simulink library "R9000_FirmwareLib"  (R2024b)
settings: Solver=VariableStepAuto  StartTime=0.0  StopTime=10.0  RelTol=1e-3
libraries: mbd_s32k3_io_library x82, mbd_s32k3_isr_library x15, mbd_s32k3_communication_library x10, mbd_s32k3_utility_library x1
(part 2 of 7)

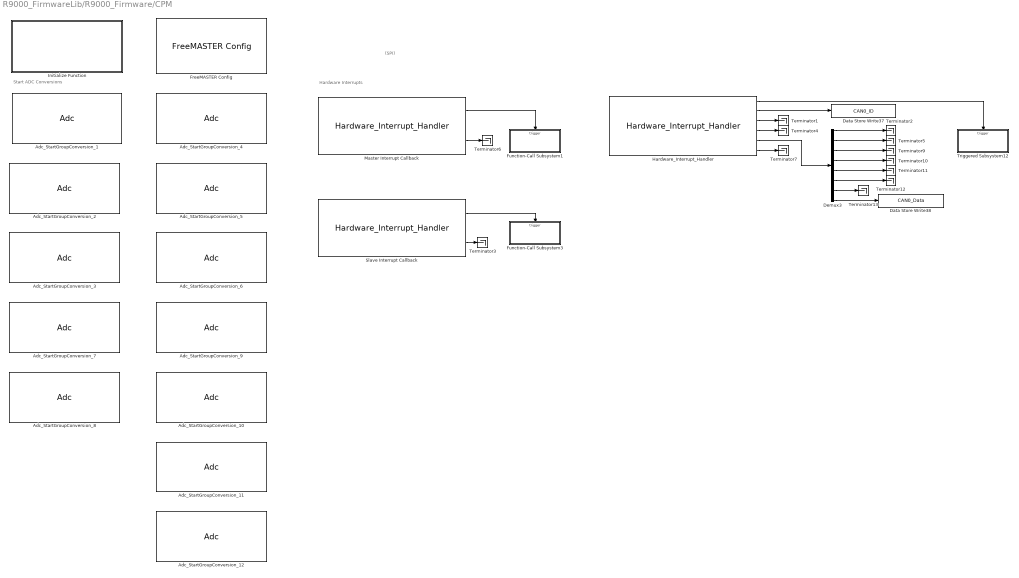
== system R9000_FirmwareLib/R9000_Firmware/CPM ==
  note: Start ADC Conversions
  note: Hardware Interrupts
  note: (SPI)
  Adc_StartGroupConversion_1 : mbd_s32k3_io_library/Adc  Priority=2  api_func=Adc_StartGroupConversion  hw_unit=AdcHwUnit_0  group=Adc0Group_0  channel=ADC_1_AI2  threshold_control=No ADC threshold control configured  power_state=ADC_FULL_POWER  ctu_unit=BctuHwUnit_0  ctu_trigger=AdcHwTrigger_0  ctu_fifo_idx=BctuResultFifos_0  text=<binary blob, 63510 chars, not shown>
      settings: SourceProductName=IO Blocks
  Adc_StartGroupConversion_10 : mbd_s32k3_io_library/Adc  Priority=2  api_func=Adc_StartGroupConversion  hw_unit=AdcHwUnit_0  group=Adc2Group_4  channel=ADC_1_AI2  threshold_control=No ADC threshold control configured  power_state=ADC_FULL_POWER  ctu_unit=BctuHwUnit_0  ctu_trigger=AdcHwTrigger_0  ctu_fifo_idx=BctuResultFifos_0  text=<binary blob, 63510 chars, not shown>
      settings: SourceProductName=IO Blocks
  Adc_StartGroupConversion_11 : mbd_s32k3_io_library/Adc  Priority=2  api_func=Adc_StartGroupConversion  hw_unit=AdcHwUnit_0  group=Adc2Group_5  channel=ADC_1_AI2  threshold_control=No ADC threshold control configured  power_state=ADC_FULL_POWER  ctu_unit=BctuHwUnit_0  ctu_trigger=AdcHwTrigger_0  ctu_fifo_idx=BctuResultFifos_0  text=<binary blob, 63510 chars, not shown>
      settings: SourceProductName=IO Blocks
  Adc_StartGroupConversion_12 : mbd_s32k3_io_library/Adc  Priority=2  api_func=Adc_StartGroupConversion  hw_unit=AdcHwUnit_0  group=Adc2Group_6  channel=ADC_1_AI2  threshold_control=No ADC threshold control configured  power_state=ADC_FULL_POWER  ctu_unit=BctuHwUnit_0  ctu_trigger=AdcHwTrigger_0  ctu_fifo_idx=BctuResultFifos_0  text=<binary blob, 63510 chars, not shown>
      settings: SourceProductName=IO Blocks
  Adc_StartGroupConversion_2 : mbd_s32k3_io_library/Adc  Priority=2  api_func=Adc_StartGroupConversion  hw_unit=AdcHwUnit_0  group=Adc0Group_1  channel=ADC_1_AI2  threshold_control=No ADC threshold control configured  power_state=ADC_FULL_POWER  ctu_unit=BctuHwUnit_0  ctu_trigger=AdcHwTrigger_0  ctu_fifo_idx=BctuResultFifos_0  text=<binary blob, 63510 chars, not shown>
      settings: SourceProductName=IO Blocks
  Adc_StartGroupConversion_3 : mbd_s32k3_io_library/Adc  Priority=2  api_func=Adc_StartGroupConversion  hw_unit=AdcHwUnit_0  group=Adc0Group_2  channel=ADC_1_AI2  threshold_control=No ADC threshold control configured  power_state=ADC_FULL_POWER  ctu_unit=BctuHwUnit_0  ctu_trigger=AdcHwTrigger_0  ctu_fifo_idx=BctuResultFifos_0  text=<binary blob, 63510 chars, not shown>
      settings: SourceProductName=IO Blocks
  Adc_StartGroupConversion_4 : mbd_s32k3_io_library/Adc  Priority=2  api_func=Adc_StartGroupConversion  hw_unit=AdcHwUnit_0  group=Adc2Group_0  channel=ADC_1_AI2  threshold_control=No ADC threshold control configured  power_state=ADC_FULL_POWER  ctu_unit=BctuHwUnit_0  ctu_trigger=AdcHwTrigger_0  ctu_fifo_idx=BctuResultFifos_0  text=<binary blob, 63510 chars, not shown>
      settings: SourceProductName=IO Blocks
  Adc_StartGroupConversion_5 : mbd_s32k3_io_library/Adc  Priority=2  api_func=Adc_StartGroupConversion  hw_unit=AdcHwUnit_0  group=Adc2Group_1  channel=ADC_1_AI2  threshold_control=No ADC threshold control configured  power_state=ADC_FULL_POWER  ctu_unit=BctuHwUnit_0  ctu_trigger=AdcHwTrigger_0  ctu_fifo_idx=BctuResultFifos_0  text=<binary blob, 63510 chars, not shown>
      settings: SourceProductName=IO Blocks
  Adc_StartGroupConversion_6 : mbd_s32k3_io_library/Adc  Priority=2  api_func=Adc_StartGroupConversion  hw_unit=AdcHwUnit_0  group=Adc2Group_2  channel=ADC_1_AI2  threshold_control=No ADC threshold control configured  power_state=ADC_FULL_POWER  ctu_unit=BctuHwUnit_0  ctu_trigger=AdcHwTrigger_0  ctu_fifo_idx=BctuResultFifos_0  text=<binary blob, 63510 chars, not shown>
      settings: SourceProductName=IO Blocks
  Adc_StartGroupConversion_7 : mbd_s32k3_io_library/Adc  Priority=2  api_func=Adc_StartGroupConversion  hw_unit=AdcHwUnit_0  group=Adc0Group_3  channel=ADC_1_AI2  threshold_control=No ADC threshold control configured  power_state=ADC_FULL_POWER  ctu_unit=BctuHwUnit_0  ctu_trigger=AdcHwTrigger_0  ctu_fifo_idx=BctuResultFifos_0  text=<binary blob, 63510 chars, not shown>
      settings: SourceProductName=IO Blocks
  Adc_StartGroupConversion_8 : mbd_s32k3_io_library/Adc  Priority=2  api_func=Adc_StartGroupConversion  hw_unit=AdcHwUnit_0  group=Adc0Group_4  channel=ADC_1_AI2  threshold_control=No ADC threshold control configured  power_state=ADC_FULL_POWER  ctu_unit=BctuHwUnit_0  ctu_trigger=AdcHwTrigger_0  ctu_fifo_idx=BctuResultFifos_0  text=<binary blob, 63510 chars, not shown>
      settings: SourceProductName=IO Blocks
  Adc_StartGroupConversion_9 : mbd_s32k3_io_library/Adc  Priority=2  api_func=Adc_StartGroupConversion  hw_unit=AdcHwUnit_0  group=Adc2Group_3  channel=ADC_1_AI2  threshold_control=No ADC threshold control configured  power_state=ADC_FULL_POWER  ctu_unit=BctuHwUnit_0  ctu_trigger=AdcHwTrigger_0  ctu_fifo_idx=BctuResultFifos_0  text=<binary blob, 63510 chars, not shown>
      settings: SourceProductName=IO Blocks
  Data Store Write37 : DataStoreWrite  DataStoreName=CAN0_ID  IndexOptions=Index vector (dialog)  Indices=1  OutputSizes=1
  Data Store Write38 : DataStoreWrite  DataStoreName=CAN0_Data  IndexOptions=Index vector (dialog)  Indices=1  OutputSizes=1
  Demux3 : Demux  Outputs=8
  FreeMASTER Config : mbd_s32k3_utility_library/FreeMASTER blocks/FreeMASTER Config  connection_type=Serial  instance=LPUART_6  baudrate=115200  isr_prio=5  cantxid=0x7aa  cantxidext=on  cantxobj=5  canrxid=0x7aa  canrxidext=on  canrxobj=5  mode=Long Interrupt  scope_use=1  scope_max_vars=8  automatic_buff_size=on  buff_size=255  default_rfifo_size=on  rfifo_size=32
      settings: SourceProductName=Utility Blocks
  Function-Call Subsystem1 : Subsystem  TreatAsAtomicUnit=on
  Function-Call Subsystem3 : Subsystem  TreatAsAtomicUnit=on
  Hardware_Interrupt_Handler : mbd_s32k3_isr_library/Hardware_Interrupt_Handler  blockCbk=0  irqGroup=Can  irqHandlers=CanIf_RxIndication  irqSim=off  text=|
      | CanConfigSet - CanConfigSet 
      | CanConfigSet/CanController - CanController_0 
      | CanConfigSet/CanController_0/CanAutoBusOffRecovery - false 
      | CanConfigSet/CanController_0/CanBusoffProcessing - POLLING 
      | CanConfigSet/CanController_0/CanControllerActivation - true 
      | CanConfigSet/CanController_0/CanControllerBaseAddress - 0 
      | CanConfigSet/CanController_0/CanControllerBaudrateConfig - CanControllerBaudrateConfig_0 
      | CanConfigSet/CanController_0/CanControllerBaudrateConfig_0/CanAdvancedSetting - false 
      | CanConfigSet/CanController_0/CanControllerBaudrateConfig_0/CanBaudrateTypeSuport - NORMAL_CBT 
      | CanConfigSet/CanController_0/CanControllerBaudrateConfig_0/CanBusLength - 40 
      | CanConfigSet/CanController_0/CanControllerBaudrateConfig_0/CanControllerBaudRate - 500.0 
      | CanConfigSet/CanController_0/CanControllerBaudrateConfig_0/CanControllerBaudRateConfigID - 0 
      | CanConfigSet/CanController_0/CanControllerBaudrateConfig_0/CanControllerFdBaudrateConfig - CanControllerFdBaudrateConfig 
      | CanConfigSet/CanController_0/CanControllerBaudrateConfig_0/CanControllerFdBaudrateConfig/CanControllerFdBaudRate - 2000 
      | CanConfigSet/CanController_0/CanControllerBaudrateConfig_0/CanControllerFdBaudrateConfig/CanControllerFdPrescaller - 2 
      | CanConfigSet/CanController_0/CanControllerBaudrateConfig_0/CanControllerFdBaudrateConfig/CanControllerFdSyncSeg - 1 
      | CanConfigSet/CanController_0/CanControllerBaudrateConfig_0/CanControllerFdBaudrateConfig/CanControllerPrescallerAlternateFd - 1 
      | CanConfigSet/CanController_0/CanControllerBaudrateConfig_0/CanControllerFdBaudrateConfig/CanControllerPropSeg - 5 
      | CanConfigSet/CanController_0/CanControllerBaudrateConfig_0/CanControllerFdBaudrateConfig/CanControllerSeg1 - 2 
      | CanConfigSet/CanController_0/CanControllerBaudrateConfig_0/CanControllerFdBaudrateConfig/CanControllerSeg2 - 2 
      | CanConfigSet/CanController_0/CanControllerBaudrateConfig_0/CanControllerFdBaudrateConfig/CanControllerSyncJumpWidth - 2 
      | CanConfigSet/CanController_0/CanControllerBaudrateConfig_0/CanControllerFdBaudrateConfig/CanControllerTxBitRateSwitch - true 
      | CanConfigSet/CanController_0/CanControllerBaudrateConfig_0/CanControllerFdBaudrateConfig/Index - 0 
      | CanConfigSet/CanController_0/CanControllerBaudrateConfig_0/CanControllerPrescaller - 2 
      | CanConfigSet/CanController_0/CanControllerBaudrateConfig_0/CanControllerPrescallerAlternate - 10 
      | CanConfigSet/CanController_0/CanControllerBaudrateConfig_0/CanControllerPropSeg - 11 
      | CanConfigSet/CanController_0/CanControllerBaudrateConfig_0/CanControllerSeg1 - 18 
      | CanConfigSet/CanController_0/CanControllerBaudrateConfig_0/CanControllerSeg2 - 10 
      | CanConfigSet/CanController_0/CanControllerBaudrateConfig_0/CanControllerSyncJumpWidth - 10 
      | CanConfigSet/CanController_0/CanControllerBaudrateConfig_0/CanControllerSyncSeg - 1 
      | CanConfigSet/CanController_0/CanControllerBaudrateConfig_0/CanPropDelayTranceiver - 150.0 
      | CanConfigSet/CanController_0/CanControllerBaudrateConfig_0/CanTxArbitrationStartDelay - 12 
      | CanConfigSet/CanController_0/CanControllerBaudrateConfig_0/Index - 0 
      | CanConfigSet/CanController_0/CanControllerDefaultBaudrate - /Can_43_FLEXCAN/Can/CanConfigSet/CanController_0/CanControllerBaudrateConfig_0 
      | CanConfigSet/CanController_0/CanControllerEdgeFilter - false 
      | CanConfigSet/CanController_0/CanControllerFdISO - true 
      | CanConfigSet/CanController_0/CanControllerId - 0 
      | CanConfigSet/CanController_0/CanControllerPrExcEn - false 
      | CanConfigSet/CanController_0/CanCpuClockRef - /Mcu/Mcu/McuModuleConfiguration/McuClockSettingConfig_0/FLEXCAN_PE_CLK0_2 
      | CanConfigSet/CanController_0/CanCpuClockRefAlternate - /Mcu/Mcu/McuModuleConfiguration/McuClockSettingConfig_0/FLEXCAN_PE_CLK0_2 
      | CanConfigSet/CanController_0/CanHwChannel - FLEXCAN_0 
      | CanConfigSet/CanController_0/CanLoopBackMode - false 
      | CanConfigSet/CanController_0/CanLowestBufferTransmittedFirst - false 
      | CanConfigSet/CanController_0/CanRamBlock - CanRamBlock 
      | CanConfigSet/CanController_0/CanRamBlock/CanRamBlockUnified - CanRamBlockUnified 
      | CanConfigSet/CanController_0/CanRamBlock/CanRamBlockUnified/CanBlockUnifiedPayloadLength - CAN_16_BYTES_PAYLOAD 
      | CanConfigSet/CanController_0/CanRamBlock/Choice - CanRamBlockUnified 
      | CanConfigSet/CanController_0/CanRamBlock/Index - 0 
      | CanConfigSet/CanController_0/CanRxFiFo - CanRxFiFo 
      | CanConfigSet/CanController_0/CanRxFiFo/CanEnhanceFiFo - CanRxFiFo 
      | CanConfigSet/CanController_0/CanRxFiFo/CanRxFiFo/CanEnhanceFiFoDmaEnable - false 
      | CanConfigSet/CanController_0/CanRxFiFo/CanRxFiFo/CanEnhancedSchemeType - MASK_FILTER_SCHEME 
      | CanConfigSet/CanController_0/CanRxFiFo/CanRxFiFo/CanFiFoDmaErrorNotif - NULL_PTR 
      | CanConfigSet/CanController_0/CanRxFiFo/CanRxFiFo/CanFiFoOverflowNotif - MBDT_FlexCAN0_FifoOverflowNotif 
      | CanConfigSet/CanController_0/CanRxFiFo/CanRxFiFo/NumberMBTransferDMA - 1 
      | CanConfigSet/CanController_0/CanRxFiFo/Choice - CanEnhanceFiFo 
      | CanConfigSet/CanController_0/CanRxFiFo/Index - 0 
      | CanConfigSet/CanController_0/CanRxProcessing - MIXED 
      | CanConfigSet/CanController_0/CanTrippleSamplingEnable - false 
      | CanConfigSet/CanController_0/CanTxProcessing - MIXED 
      | CanConfigSet/CanController_0/CanWakeupFunctionalityAPI - false 
      | CanConfigSet/CanController_0/CanWakeupProcessing - POLLING 
      | CanConfigSet/CanController_0/CanWakeupSupport - false 
      | CanConfigSet/CanController_0/Index - 0 
      | CanConfigSet/CanHardwareObject - CanHardwareObject_Can0_Rx_Ext_Interrupt CanHardwareObject_Can0_Rx_FIFO CanHardwareObject_Can0_Rx_Std_Polling CanHardwareObject_Can0_Tx_Interrupt CanHardwareObject_Can0_Tx_Polling 
      | CanConfigSet/CanHardwareObject_Can0_Rx_Ext_Interrupt/CanControllerRef - /Can_43_FLEXCAN/Can/CanConfigSet/CanController_0 
      | CanConfigSet/CanHardwareObject_Can0_Rx_Ext_Interrupt/CanHandleType - BASIC 
      | CanConfigSet/CanHardwareObject_Can0_Rx_Ext_Interrupt/CanHardwareObjectUsesPolling - false 
      | CanConfigSet/CanHardwareObject_Can0_Rx_Ext_Interrupt/CanHwFilter - CanHwFilter_0 
      | CanConfigSet/CanHardwareObject_Can0_Rx_Ext_Interrupt/CanHwFilter_0/CanHwFilterCode - 0 
      | CanConfigSet/CanHardwareObject_Can0_Rx_Ext_Interrupt/CanHwFilter_0/CanHwFilterIDE - false 
      | CanConfigSet/CanHardwareObject_Can0_Rx_Ext_Interrupt/CanHwFilter_0/CanHwFilterMask - 0 
      | CanConfigSet/CanHardwareObject_Can0_Rx_Ext_Interrupt/CanHwFilter_0/Index - 0 
      | CanConfigSet/CanHardwareObject_Can0_Rx_Ext_Interrupt/CanHwObjectCount - 1 
      | CanConfigSet/CanHardwareObject_Can0_Rx_Ext_Interrupt/CanIdType - EXTENDED 
      | CanConfigSet/CanHardwareObject_Can0_Rx_Ext_Interrupt/CanObjectId - 2 
      | CanConfigSet/CanHardwareObject_Can0_Rx_Ext_Interrupt/CanObjectType - RECEIVE 
      | CanConfigSet/CanHardwareObject_Can0_Rx_Ext_Interrupt/Index - 2 
      | CanConfigSet/CanHardwareObject_Can0_Rx_FIFO/CanControllerRef - /Can_43_FLEXCAN/Can/CanConfigSet/CanController_0 
      | CanConfigSet/CanHardwareObject_Can0_Rx_FIFO/CanHandleType - BASIC 
      | CanConfigSet/CanHardwareObject_Can0_Rx_FIFO/CanHardwareObjectUsesPolling - false 
      | CanConfigSet/CanHardwareObject_Can0_Rx_FIFO/CanHwFilter - CanHwFilter_0 CanHwFilter_1 CanHwFilter_2 CanHwFilter_3 CanHwFilter_4 CanHwFilter_5 CanHwFilter_6 CanHwFilter_7 
      | CanConfigSet/CanHardwareObject_Can0_Rx_FIFO/CanHwFilter_0/CanHwFilterCode - 1008 
      | CanConfigSet/CanHardwareObject_Can0_Rx_FIFO/CanHwFilter_0/CanHwFilterIDE - false 
      | CanConfigSet/CanHardwareObject_Can0_Rx_FIFO/CanHwFilter_0/CanHwFilterMask - 536870911 
      | CanConfigSet/CanHardwareObject_Can0_Rx_FIFO/CanHwFilter_0/Index - 0 
      | CanConfigSet/CanHardwareObject_Can0_Rx_FIFO/CanHwFilter_1/CanHwFilterCode - 1009 
      | CanConfigSet/CanHardwareObject_Can0_Rx_FIFO/CanHwFilter_1/CanHwFilterIDE - false 
      | CanConfigSet/CanHardwareObject_Can0_Rx_FIFO/CanHwFilter_1/CanHwFilterMask - 536870911 
      | CanConfigSet/CanHardwareObject_Can0_Rx_FIFO/CanHwFilter_1/Index - 1 
      | CanConfigSet/CanHardwareObject_Can0_Rx_FIFO/CanHwFilter_2/CanHwFilterCode - 1010 
      | CanConfigSet/CanHardwareObject_Can0_Rx_FIFO/CanHwFilter_2/CanHwFilterIDE - false 
      | CanConfigSet/CanHardwareObject_Can0_Rx_FIFO/CanHwFilter_2/CanHwFilterMask - 536870911 
      | CanConfigSet/CanHardwareObject_Can0_Rx_FIFO/CanHwFilter_2/Index - 2 
      | CanConfigSet/CanHardwareObject_Can0_Rx_FIFO/CanHwFilter_3/CanHwFilterCode - 1011 
      | CanConfigSet/CanHardwareObject_Can0_Rx_FIFO/CanHwFilter_3/CanHwFilterIDE - false 
      | CanConfigSet/CanHardwareObject_Can0_Rx_FIFO/CanHwFilter_3/CanHwFilterMask - 536870911 
      | CanConfigSet/CanHardwareObject_Can0_Rx_FIFO/CanHwFilter_3/Index - 3 
      | CanConfigSet/CanHardwareObject_Can0_Rx_FIFO/CanHwFilter_4/CanHwFilterCode - 1012 
      | CanConfigSet/CanHardwareObject_Can0_Rx_FIFO/CanHwFilter_4/CanHwFilterIDE - false 
      | CanConfigSet/CanHardwareObject_Can0_Rx_FIFO/CanHwFilter_4/CanHwFilterMask - 536870911 
      | CanConfigSet/CanHardwareObject_Can0_Rx_FIFO/CanHwFilter_4/Index - 4 
      | CanConfigSet/CanHardwareObject_Can0_Rx_FIFO/CanHwFilter_5/CanHwFilterCode - 1013 
      | CanConfigSet/CanHardwareObject_Can0_Rx_FIFO/CanHwFilter_5/CanHwFilterIDE - false 
      | CanConfigSet/CanHardwareObject_Can0_Rx_FIFO/CanHwFilter_5/CanHwFilterMask - 536870911 
      | CanConfigSet/CanHardwareObject_Can0_Rx_FIFO/CanHwFilter_5/Index - 5 
      | CanConfigSet/CanHardwareObject_Can0_Rx_FIFO/CanHwFilter_6/CanHwFilterCode - 1014 
      | CanConfigSet/CanHardwareObject_Can0_Rx_FIFO/CanHwFilter_6/CanHwFilterIDE - false 
      | CanConfigSet/CanHardwareObject_Can0_Rx_FIFO/CanHwFilter_6/CanHwFilterMask - 536870911 
      | CanConfigSet/CanHardwareObject_Can0_Rx_FIFO/CanHwFilter_6/Index - 6 
      | CanConfigSet/CanHardwareObject_Can0_Rx_FIFO/CanHwFilter_7/CanHwFilterCode - 1015 
      | CanConfigSet/CanHardwareObject_Can0_Rx_FIFO/CanHwFilter_7/CanHwFilterIDE - false 
      | CanConfigSet/CanHardwareObject_Can0_Rx_FIFO/CanHwFilter_7/CanHwFilterMask - 536870911 
      | CanConfigSet/CanHardwareObject_Can0_Rx_FIFO/CanHwFilter_7/Index - 7 
      | CanConfigSet/CanHardwareObject_Can0_Rx_FIFO/CanHwObjectCount - 6 
      | CanConfigSet/CanHardwareObject_Can0_Rx_FIFO/CanIdType - EXTENDED 
      | CanConfigSet/CanHardwareObject_Can0_Rx_FIFO/CanObjectId - 0 
      | CanConfigSet/CanHardwareObject_Can0_Rx_FIFO/CanObjectType - RECEIVE 
      | CanConfigSet/CanHardwareObject_Can0_Rx_FIFO/Index - 0 
      | CanConfigSet/CanHardwareObject_Can0_Rx_Std_Polling/CanControllerRef - /Can_43_FLEXCAN/Can/CanConfigSet/CanController_0 
      | CanConfigSet/CanHardwareObject_Can0_Rx_Std_Polling/CanHandleType - BASIC 
      | CanConfigSet/CanHardwareObject_Can0_Rx_Std_Polling/CanHardwareObjectUsesPolling - true 
      | CanConfigSet/CanHardwareObject_Can0_Rx_Std_Polling/CanHwFilter - CanHwFilter_0 
      | CanConfigSet/CanHardwareObject_Can0_Rx_Std_Polling/CanHwFilter_0/CanHwFilterCode - 0 
      | CanConfigSet/CanHardwareObject_Can0_Rx_Std_Polling/CanHwFilter_0/CanHwFilterIDE - false 
      | CanConfigSet/CanHardwareObject_Can0_Rx_Std_Polling/CanHwFilter_0/CanHwFilterMask - 0 
      | CanConfigSet/CanHardwareObject_Can0_Rx_Std_Polling/CanHwFilter_0/Index - 0 
      | CanConfigSet/CanHardwareObject_Can0_Rx_Std_Polling/CanHwObjectCount - 1 
      | CanConfigSet/CanHardwareObject_Can0_Rx_Std_Polling/CanIdType - STANDARD 
      | CanConfigSet/CanHardwareObject_Can0_Rx_Std_Polling/CanMainFunctionRWPeriodRef - /Can_43_FLEXCAN/Can/CanGeneral/CanMainFunctionRWPeriods_0 
      | CanConfigSet/CanHardwareObject_Can0_Rx_Std_Polling/CanObjectId - 1 
      | CanConfigSet/CanHardwareObject_Can0_Rx_Std_Polling/CanObjectType - RECEIVE 
      | CanConfigSet/CanHardwareObject_Can0_Rx_Std_Polling/Index - 1 
      | CanConfigSet/CanHardwareObject_Can0_Tx_Interrupt/CanControllerRef - /Can_43_FLEXCAN/Can/CanConfigSet/CanController_0 
      | CanConfigSet/CanHardwareObject_Can0_Tx_Interrupt/CanHandleType - BASIC 
      | CanConfigSet/CanHardwareObject_Can0_Tx_Interrupt/CanHardwareObjectUsesPolling - false 
      | CanConfigSet/CanHardwareObject_Can0_Tx_Interrupt/CanHwObjectCount - 1 
      | CanConfigSet/CanHardwareObject_Can0_Tx_Interrupt/CanIdType - MIXED 
      | CanConfigSet/CanHardwareObject_Can0_Tx_Interrupt/CanObjectId - 4 
      | CanConfigSet/CanHardwareObject_Can0_Tx_Interrupt/CanObjectType - TRANSMIT 
      | CanConfigSet/CanHardwareObject_Can0_Tx_Interrupt/Index - 4 
      | CanConfigSet/CanHardwareObject_Can0_Tx_Polling/CanControllerRef - /Can_43_FLEXCAN/Can/CanConfigSet/CanController_0 
      | CanConfigSet/CanHardwareObject_Can0_Tx_Polling/CanHandleType - BASIC 
      | CanConfigSet/CanHardwareObject_Can0_Tx_Polling/CanHardwareObjectUsesPolling - true 
      | CanConfigSet/CanHardwareObject_Can0_Tx_Polling/CanHwObjectCount - 1 
      | CanConfigSet/CanHardwareObject_Can0_Tx_Polling/CanIdType - MIXED 
      | CanConfigSet/CanHardwareObject_Can0_Tx_Polling/CanMainFunctionRWPeriodRef - /Can_43_FLEXCAN/Can/CanGeneral/CanMainFunctionRWPeriods_0 
      | CanConfigSet/CanHardwareObject_Can0_Tx_Polling/CanObjectId - 3 
      | CanConfigSet/CanHardwareObject_Can0_Tx_Polling/CanObjectType - TRANSMIT 
      | CanConfigSet/CanHardwareObject_Can0_Tx_Polling/Index - 3 
      | CanGeneral - CanGeneral 
      | CanGeneral/CanApiEnableMbAbort - true 
      | CanGeneral/CanDevErrorDetect - true 
      | CanGeneral/CanEnableDualClockMode - false 
      | CanGeneral/CanEnableSecurityEventReporting - false 
      | CanGeneral/CanEnableUserModeSupport - false 
      | CanGeneral/CanGlobalTimeSupport - false 
      | CanGeneral/CanIndex - 0 
      | CanGeneral/CanMainFunctionModePeriod - 0.001 
      | CanGeneral/CanMainFunctionRWPeriods - CanMainFunctionRWPeriods_0 
      | CanGeneral/CanMainFunctionRWPeriods_0/CanMainFunctionPeriod - 0.001 
      | CanGeneral/CanMainFunctionRWPeriods_0/Index - 0 
      | CanGeneral/CanMultiPartitionSupport - false 
      | CanGeneral/CanMultiplexedTransmission - true 
      | CanGeneral/CanSetBaudrateApi - true 
      | CanGeneral/CanTimeoutDuration - 1.0 
      | CanGeneral/CanTimeoutMethod - OSIF_COUNTER_DUMMY 
      | CanGeneral/CanVersionInfoApi - true 
      | CommonPublishedInformation - CommonPublishedInformation 
      | CommonPublishedInformation/ArReleaseMajorVersion - 4 
      | CommonPublishedInformation/ArReleaseMinorVersion - 7 
      | CommonPublishedInformation/ArReleaseRevisionVersion - 0 
      | CommonPublishedInformation/ModuleId - 80 
      | CommonPublishedInformation/SwMajorVersion - 3 
      | CommonPublishedInformation/SwMinorVersion - 0 
      | CommonPublishedInformation/SwPatchVersion - 0 
      | CommonPublishedInformation/VendorApiInfix - FLEXCAN 
      | CommonPublishedInformation/VendorId - 43 
      | IMPLEMENTATION-CONFIG-VARIANT - VARIANT-POST-BUILD 
      | POST_BUILD_VARIANT_USED - true
      settings: SourceProductName=ISR Blocks
  Initialize Function : Subsystem  TreatAsAtomicUnit=on
  Master Interrupt Callback : mbd_s32k3_isr_library/Hardware_Interrupt_Handler  blockCbk=0  irqGroup=Spi  irqHandlers=MBDT_SPI_end_job0_callback  irqSim=off  text=|
      | CommonPublishedInformation - CommonPublishedInformation 
      | CommonPublishedInformation/ArReleaseMajorVersion - 4 
      | CommonPublishedInformation/ArReleaseMinorVersion - 4 
      | CommonPublishedInformation/ArReleaseRevisionVersion - 0 
      | CommonPublishedInformation/ModuleId - 83 
      | CommonPublishedInformation/SwMajorVersion - 2 
      | CommonPublishedInformation/SwMinorVersion - 0 
      | CommonPublishedInformation/SwPatchVersion - 0 
      | CommonPublishedInformation/VendorId - 43 
      | IMPLEMENTATION-CONFIG-VARIANT - VARIANT-POST-BUILD 
      | POST_BUILD_VARIANT_USED - true 
      | SpiAutosarExt - SpiAutosarExt 
      | SpiAutosarExt/SpiAllowBigSizeCollections - false 
      | SpiAutosarExt/SpiDisableDemReportErrorStatus - false 
      | SpiAutosarExt/SpiEnableDmaContMemTransferSupport - false 
      | SpiAutosarExt/SpiEnableDmaFastTransferSupport - false 
      | SpiAutosarExt/SpiEnableHWUnitAsyncMode - false 
      | SpiAutosarExt/SpiEnableUserModeSupport - false 
      | SpiAutosarExt/SpiFlexioEnable - false 
      | SpiAutosarExt/SpiHalfDuplexModeSupport - false 
      | SpiAutosarExt/SpiJobStartNotificationEnable - false 
      | SpiAutosarExt/SpiUseLegacyEBLength - false 
      | SpiDriver - SpiDriver 
      | SpiDriver/SpiChannel - SpiChannel_0 SpiChannel_1 
      | SpiDriver/SpiChannel_0/Index - 0 
      | SpiDriver/SpiChannel_0/SpiByteSwapTransfer - false 
      | SpiDriver/SpiChannel_0/SpiChannelId - 0 
      | SpiDriver/SpiChannel_0/SpiChannelType - IB 
      | SpiDriver/SpiChannel_0/SpiDataWidth - 32 
      | SpiDriver/SpiChannel_0/SpiEbMaxLength - 1000 
      | SpiDriver/SpiChannel_0/SpiIbNBuffers - 4 
      | SpiDriver/SpiChannel_0/SpiTransferStart - MSB 
      | SpiDriver/SpiChannel_1/Index - 1 
      | SpiDriver/SpiChannel_1/SpiByteSwapTransfer - false 
      | SpiDriver/SpiChannel_1/SpiChannelId - 1 
      | SpiDriver/SpiChannel_1/SpiChannelType - IB 
      | SpiDriver/SpiChannel_1/SpiDataWidth - 8 
      | SpiDriver/SpiChannel_1/SpiEbMaxLength - 1000 
      | SpiDriver/SpiChannel_1/SpiIbNBuffers - 1 
      | SpiDriver/SpiChannel_1/SpiTransferStart - MSB 
      | SpiDriver/SpiExternalDevice - SpiExternalDevice_0 SpiExternalDevice_1 
      | SpiDriver/SpiExternalDevice_0/Index - 0 
      | SpiDriver/SpiExternalDevice_0/SpiBaudrate - 1000000.0 
      | SpiDriver/SpiExternalDevice_0/SpiCsBehavior - CS_KEEP_ASSERTED 
      | SpiDriver/SpiExternalDevice_0/SpiCsIdentifier - PCS0 
      | SpiDriver/SpiExternalDevice_0/SpiCsPolarity - LOW 
      | SpiDriver/SpiExternalDevice_0/SpiCsSelection - CS_VIA_PERIPHERAL_ENGINE 
      | SpiDriver/SpiExternalDevice_0/SpiDataShiftEdge - LEADING 
      | SpiDriver/SpiExternalDevice_0/SpiDeviceHalfDuplexSupport - false 
      | SpiDriver/SpiExternalDevice_0/SpiEnableCs - true 
      | SpiDriver/SpiExternalDevice_0/SpiHalfDuplexPinSelect - HALF_DUPLEX_SIN 
      | SpiDriver/SpiExternalDevice_0/SpiHostRequest - DISABLE 
      | SpiDriver/SpiExternalDevice_0/SpiHwUnit - CSIB0 
      | SpiDriver/SpiExternalDevice_0/SpiShiftClockIdleLevel - LOW 
      | SpiDriver/SpiExternalDevice_0/SpiTimeClk2Cs - 2.0E-7 
      | SpiDriver/SpiExternalDevice_0/SpiTimeCs2Clk - 2.0E-7 
      | SpiDriver/SpiExternalDevice_0/SpiTimeCs2Cs - 4.0E-7 
      | SpiDriver/SpiExternalDevice_0/SpiTransferWidth - TRANSFER_1_BIT 
      | SpiDriver/SpiExternalDevice_0/SpiUseBaudrateConfig - false 
      | SpiDriver/SpiExternalDevice_1/Index - 1 
      | SpiDriver/SpiExternalDevice_1/SpiBaudrate - 500000.0 
      | SpiDriver/SpiExternalDevice_1/SpiCsBehavior - CS_KEEP_ASSERTED 
      | SpiDriver/SpiExternalDevice_1/SpiCsIdentifier - PCS3 
      | SpiDriver/SpiExternalDevice_1/SpiCsPolarity - LOW 
      | SpiDriver/SpiExternalDevice_1/SpiCsSelection - CS_VIA_PERIPHERAL_ENGINE 
      | SpiDriver/SpiExternalDevice_1/SpiDataShiftEdge - LEADING 
      | SpiDriver/SpiExternalDevice_1/SpiDeviceHalfDuplexSupport - false 
      | SpiDriver/SpiExternalDevice_1/SpiEnableCs - true 
      | SpiDriver/SpiExternalDevice_1/SpiHalfDuplexPinSelect - HALF_DUPLEX_SIN 
      | SpiDriver/SpiExternalDevice_1/SpiHostRequest - DISABLE 
      | SpiDriver/SpiExternalDevice_1/SpiHwUnit - CSIB1 
      | SpiDriver/SpiExternalDevice_1/SpiShiftClockIdleLevel - LOW 
      | SpiDriver/SpiExternalDevice_1/SpiTimeClk2Cs - 2.0E-7 
      | SpiDriver/SpiExternalDevice_1/SpiTimeCs2Clk - 2.0E-7 
      | SpiDriver/SpiExternalDevice_1/SpiTimeCs2Cs - 4.0E-7 
      | SpiDriver/SpiExternalDevice_1/SpiTransferWidth - TRANSFER_1_BIT 
      | SpiDriver/SpiExternalDevice_1/SpiUseBaudrateConfig - false 
      | SpiDriver/SpiJob - SpiJob_0 SpiJob_1 
      | SpiDriver/SpiJob_0/Index - 0 
      | SpiDriver/SpiJob_0/SpiChannelList - SpiChannelList_0 
      | SpiDriver/SpiJob_0/SpiChannelList_0/Index - 0 
      | SpiDriver/SpiJob_0/SpiChannelList_0/SpiChannelAssignment - /Spi/Spi/SpiDriver/SpiChannel_0 
      | SpiDriver/SpiJob_0/SpiChannelList_0/SpiChannelIndex - 0 
      | SpiDriver/SpiJob_0/SpiDeviceAssignment - /Spi/Spi/SpiDriver/SpiExternalDevice_0 
      | SpiDriver/SpiJob_0/SpiJobEndNotification - MBDT_SPI_end_job0_callback 
      | SpiDriver/SpiJob_0/SpiJobId - 0 
      | SpiDriver/SpiJob_0/SpiJobPriority - 0 
      | SpiDriver/SpiJob_1/Index - 1 
      | SpiDriver/SpiJob_1/SpiChannelList - SpiChannelList_0 
      | SpiDriver/SpiJob_1/SpiChannelList_0/Index - 0 
      | SpiDriver/SpiJob_1/SpiChannelList_0/SpiChannelAssignment - /Spi/Spi/SpiDriver/SpiChannel_1 
      | SpiDriver/SpiJob_1/SpiChannelList_0/SpiChannelIndex - 0 
      | SpiDriver/SpiJob_1/SpiDeviceAssignment - /Spi/Spi/SpiDriver/SpiExternalDevice_1 
      | SpiDriver/SpiJob_1/SpiJobId - 1 
      | SpiDriver/SpiJob_1/SpiJobPriority - 0 
      | SpiDriver/SpiSequence - SpiSequence_0 SpiSequence_1 
      | SpiDriver/SpiSequence_0/Index - 0 
      | SpiDriver/SpiSequence_0/SpiDmaContMemTransferSequenceEnable - false 
      | SpiDriver/SpiSequence_0/SpiEnableDmaFastTransfer - false 
      | SpiDriver/SpiSequence_0/SpiInterruptibleSequence - false 
      | SpiDriver/SpiSequence_0/SpiJobAssignment - /Spi/Spi/SpiDriver/SpiJob_0 
      | SpiDriver/SpiSequence_0/SpiSequenceId - 0 
      | SpiDriver/SpiSequence_1/Index - 1 
      | SpiDriver/SpiSequence_1/SpiDmaContMemTransferSequenceEnable - false 
      | SpiDriver/SpiSequence_1/SpiEnableDmaFastTransfer - false 
      | SpiDriver/SpiSequence_1/SpiInterruptibleSequence - false 
      | SpiDriver/SpiSequence_1/SpiJobAssignment - /Spi/Spi/SpiDriver/SpiJob_1 
      | SpiDriver/SpiSequence_1/SpiSeqEndNotification - MBDT_SPI_end_sequence1_callback 
      | SpiDriver/SpiSequence_1/SpiSequenceId - 1 
      | SpiGeneral - SpiGeneral 
      | SpiGeneral/SpiCancelApi - true 
      | SpiGeneral/SpiChannelBuffersAllowed - 2 
      | SpiGeneral/SpiDevErrorDetect - true 
      | SpiGeneral/SpiGlobalDmaEnable - false 
      | SpiGeneral/SpiHwStatusApi - true 
      | SpiGeneral/SpiInterruptibleSeqAllowed - true 
      | SpiGeneral/SpiLevelDelivered - 2 
      | SpiGeneral/SpiMultipartitionSupport - false 
      | SpiGeneral/SpiPhyUnit - SpiPhyUnit_0 SpiPhyUnit_1 
      | SpiGeneral/SpiPhyUnit_0/Index - 0 
      | SpiGeneral/SpiPhyUnit_0/SpiPhyUnitAsyncUseDma - false 
      | SpiGeneral/SpiPhyUnit_0/SpiPhyUnitClockRef - /Mcu/Mcu/McuModuleConfiguration/McuClockSettingConfig_0/SPI_CLK 
      | SpiGeneral/SpiPhyUnit_0/SpiPhyUnitMapping - LPSPI_2 
      | SpiGeneral/SpiPhyUnit_0/SpiPhyUnitMode - SPI_MASTER 
      | SpiGeneral/SpiPhyUnit_0/SpiPinConfiguration - 0 
      | SpiGeneral/SpiPhyUnit_0/SpiSamplePoint - 0 
      | SpiGeneral/SpiPhyUnit_1/Index - 1 
      | SpiGeneral/SpiPhyUnit_1/SpiPhyUnitAsyncUseDma - false 
      | SpiGeneral/SpiPhyUnit_1/SpiPhyUnitClockRef - /Mcu/Mcu/McuModuleConfiguration/McuClockSettingConfig_0/SPI_CLK 
      | SpiGeneral/SpiPhyUnit_1/SpiPhyUnitMapping - LPSPI_1 
      | SpiGeneral/SpiPhyUnit_1/SpiPhyUnitMode - SPI_SLAVE 
      | SpiGeneral/SpiPhyUnit_1/SpiPinConfiguration - 0 
      | SpiGeneral/SpiPhyUnit_1/SpiSamplePoint - 0 
      | SpiGeneral/SpiSupportConcurrentSyncTransmit - false 
      | SpiGeneral/SpiTimeoutMethod - OSIF_COUNTER_DUMMY 
      | SpiGeneral/SpiTransmitTimeout - 50000 
      | SpiGeneral/SpiVersionInfoApi - true 
      | SpiPublishedInformation - SpiPublishedInformation 
      | SpiPublishedInformation/SpiMaxHwUnit - 0
      settings: SourceProductName=ISR Blocks
  Slave Interrupt Callback : mbd_s32k3_isr_library/Hardware_Interrupt_Handler  blockCbk=0  irqGroup=Spi  irqHandlers=MBDT_SPI_end_sequence1_callback  irqSim=off  text=|
      | CommonPublishedInformation - CommonPublishedInformation 
      | CommonPublishedInformation/ArReleaseMajorVersion - 4 
      | CommonPublishedInformation/ArReleaseMinorVersion - 4 
      | CommonPublishedInformation/ArReleaseRevisionVersion - 0 
      | CommonPublishedInformation/ModuleId - 83 
      | CommonPublishedInformation/SwMajorVersion - 2 
      | CommonPublishedInformation/SwMinorVersion - 0 
      | CommonPublishedInformation/SwPatchVersion - 0 
      | CommonPublishedInformation/VendorId - 43 
      | IMPLEMENTATION-CONFIG-VARIANT - VARIANT-POST-BUILD 
      | POST_BUILD_VARIANT_USED - true 
      | SpiAutosarExt - SpiAutosarExt 
      | SpiAutosarExt/SpiAllowBigSizeCollections - false 
      | SpiAutosarExt/SpiDisableDemReportErrorStatus - false 
      | SpiAutosarExt/SpiEnableDmaContMemTransferSupport - false 
      | SpiAutosarExt/SpiEnableDmaFastTransferSupport - false 
      | SpiAutosarExt/SpiEnableHWUnitAsyncMode - false 
      | SpiAutosarExt/SpiEnableUserModeSupport - false 
      | SpiAutosarExt/SpiFlexioEnable - false 
      | SpiAutosarExt/SpiHalfDuplexModeSupport - false 
      | SpiAutosarExt/SpiJobStartNotificationEnable - false 
      | SpiAutosarExt/SpiUseLegacyEBLength - false 
      | SpiDriver - SpiDriver 
      | SpiDriver/SpiChannel - SpiChannel_0 SpiChannel_1 
      | SpiDriver/SpiChannel_0/Index - 0 
      | SpiDriver/SpiChannel_0/SpiByteSwapTransfer - false 
      | SpiDriver/SpiChannel_0/SpiChannelId - 0 
      | SpiDriver/SpiChannel_0/SpiChannelType - IB 
      | SpiDriver/SpiChannel_0/SpiDataWidth - 32 
      | SpiDriver/SpiChannel_0/SpiEbMaxLength - 1000 
      | SpiDriver/SpiChannel_0/SpiIbNBuffers - 4 
      | SpiDriver/SpiChannel_0/SpiTransferStart - MSB 
      | SpiDriver/SpiChannel_1/Index - 1 
      | SpiDriver/SpiChannel_1/SpiByteSwapTransfer - false 
      | SpiDriver/SpiChannel_1/SpiChannelId - 1 
      | SpiDriver/SpiChannel_1/SpiChannelType - IB 
      | SpiDriver/SpiChannel_1/SpiDataWidth - 8 
      | SpiDriver/SpiChannel_1/SpiEbMaxLength - 1000 
      | SpiDriver/SpiChannel_1/SpiIbNBuffers - 1 
      | SpiDriver/SpiChannel_1/SpiTransferStart - MSB 
      | SpiDriver/SpiExternalDevice - SpiExternalDevice_0 SpiExternalDevice_1 
      | SpiDriver/SpiExternalDevice_0/Index - 0 
      | SpiDriver/SpiExternalDevice_0/SpiBaudrate - 1000000.0 
      | SpiDriver/SpiExternalDevice_0/SpiCsBehavior - CS_KEEP_ASSERTED 
      | SpiDriver/SpiExternalDevice_0/SpiCsIdentifier - PCS0 
      | SpiDriver/SpiExternalDevice_0/SpiCsPolarity - LOW 
      | SpiDriver/SpiExternalDevice_0/SpiCsSelection - CS_VIA_PERIPHERAL_ENGINE 
      | SpiDriver/SpiExternalDevice_0/SpiDataShiftEdge - LEADING 
      | SpiDriver/SpiExternalDevice_0/SpiDeviceHalfDuplexSupport - false 
      | SpiDriver/SpiExternalDevice_0/SpiEnableCs - true 
      | SpiDriver/SpiExternalDevice_0/SpiHalfDuplexPinSelect - HALF_DUPLEX_SIN 
      | SpiDriver/SpiExternalDevice_0/SpiHostRequest - DISABLE 
      | SpiDriver/SpiExternalDevice_0/SpiHwUnit - CSIB0 
      | SpiDriver/SpiExternalDevice_0/SpiShiftClockIdleLevel - LOW 
      | SpiDriver/SpiExternalDevice_0/SpiTimeClk2Cs - 2.0E-7 
      | SpiDriver/SpiExternalDevice_0/SpiTimeCs2Clk - 2.0E-7 
      | SpiDriver/SpiExternalDevice_0/SpiTimeCs2Cs - 4.0E-7 
      | SpiDriver/SpiExternalDevice_0/SpiTransferWidth - TRANSFER_1_BIT 
      | SpiDriver/SpiExternalDevice_0/SpiUseBaudrateConfig - false 
      | SpiDriver/SpiExternalDevice_1/Index - 1 
      | SpiDriver/SpiExternalDevice_1/SpiBaudrate - 500000.0 
      | SpiDriver/SpiExternalDevice_1/SpiCsBehavior - CS_KEEP_ASSERTED 
      | SpiDriver/SpiExternalDevice_1/SpiCsIdentifier - PCS3 
      | SpiDriver/SpiExternalDevice_1/SpiCsPolarity - LOW 
      | SpiDriver/SpiExternalDevice_1/SpiCsSelection - CS_VIA_PERIPHERAL_ENGINE 
      | SpiDriver/SpiExternalDevice_1/SpiDataShiftEdge - LEADING 
      | SpiDriver/SpiExternalDevice_1/SpiDeviceHalfDuplexSupport - false 
      | SpiDriver/SpiExternalDevice_1/SpiEnableCs - true 
      | SpiDriver/SpiExternalDevice_1/SpiHalfDuplexPinSelect - HALF_DUPLEX_SIN 
      | SpiDriver/SpiExternalDevice_1/SpiHostRequest - DISABLE 
      | SpiDriver/SpiExternalDevice_1/SpiHwUnit - CSIB1 
      | SpiDriver/SpiExternalDevice_1/SpiShiftClockIdleLevel - LOW 
      | SpiDriver/SpiExternalDevice_1/SpiTimeClk2Cs - 2.0E-7 
      | SpiDriver/SpiExternalDevice_1/SpiTimeCs2Clk - 2.0E-7 
      | SpiDriver/SpiExternalDevice_1/SpiTimeCs2Cs - 4.0E-7 
      | SpiDriver/SpiExternalDevice_1/SpiTransferWidth - TRANSFER_1_BIT 
      | SpiDriver/SpiExternalDevice_1/SpiUseBaudrateConfig - false 
      | SpiDriver/SpiJob - SpiJob_0 SpiJob_1 
      | SpiDriver/SpiJob_0/Index - 0 
      | SpiDriver/SpiJob_0/SpiChannelList - SpiChannelList_0 
      | SpiDriver/SpiJob_0/SpiChannelList_0/Index - 0 
      | SpiDriver/SpiJob_0/SpiChannelList_0/SpiChannelAssignment - /Spi/Spi/SpiDriver/SpiChannel_0 
      | SpiDriver/SpiJob_0/SpiChannelList_0/SpiChannelIndex - 0 
      | SpiDriver/SpiJob_0/SpiDeviceAssignment - /Spi/Spi/SpiDriver/SpiExternalDevice_0 
      | SpiDriver/SpiJob_0/SpiJobEndNotification - MBDT_SPI_end_job0_callback 
      | SpiDriver/SpiJob_0/SpiJobId - 0 
      | SpiDriver/SpiJob_0/SpiJobPriority - 0 
      | SpiDriver/SpiJob_1/Index - 1 
      | SpiDriver/SpiJob_1/SpiChannelList - SpiChannelList_0 
      | SpiDriver/SpiJob_1/SpiChannelList_0/Index - 0 
      | SpiDriver/SpiJob_1/SpiChannelList_0/SpiChannelAssignment - /Spi/Spi/SpiDriver/SpiChannel_1 
      | SpiDriver/SpiJob_1/SpiChannelList_0/SpiChannelIndex - 0 
      | SpiDriver/SpiJob_1/SpiDeviceAssignment - /Spi/Spi/SpiDriver/SpiExternalDevice_1 
      | SpiDriver/SpiJob_1/SpiJobId - 1 
      | SpiDriver/SpiJob_1/SpiJobPriority - 0 
      | SpiDriver/SpiSequence - SpiSequence_0 SpiSequence_1 
      | SpiDriver/SpiSequence_0/Index - 0 
      | SpiDriver/SpiSequence_0/SpiDmaContMemTransferSequenceEnable - false 
      | SpiDriver/SpiSequence_0/SpiEnableDmaFastTransfer - false 
      | SpiDriver/SpiSequence_0/SpiInterruptibleSequence - false 
      | SpiDriver/SpiSequence_0/SpiJobAssignment - /Spi/Spi/SpiDriver/SpiJob_0 
      | SpiDriver/SpiSequence_0/SpiSequenceId - 0 
      | SpiDriver/SpiSequence_1/Index - 1 
      | SpiDriver/SpiSequence_1/SpiDmaContMemTransferSequenceEnable - false 
      | SpiDriver/SpiSequence_1/SpiEnableDmaFastTransfer - false 
      | SpiDriver/SpiSequence_1/SpiInterruptibleSequence - false 
      | SpiDriver/SpiSequence_1/SpiJobAssignment - /Spi/Spi/SpiDriver/SpiJob_1 
      | SpiDriver/SpiSequence_1/SpiSeqEndNotification - MBDT_SPI_end_sequence1_callback 
      | SpiDriver/SpiSequence_1/SpiSequenceId - 1 
      | SpiGeneral - SpiGeneral 
      | SpiGeneral/SpiCancelApi - true 
      | SpiGeneral/SpiChannelBuffersAllowed - 2 
      | SpiGeneral/SpiDevErrorDetect - true 
      | SpiGeneral/SpiGlobalDmaEnable - false 
      | SpiGeneral/SpiHwStatusApi - true 
      | SpiGeneral/SpiInterruptibleSeqAllowed - true 
      | SpiGeneral/SpiLevelDelivered - 2 
      | SpiGeneral/SpiMultipartitionSupport - false 
      | SpiGeneral/SpiPhyUnit - SpiPhyUnit_0 SpiPhyUnit_1 
      | SpiGeneral/SpiPhyUnit_0/Index - 0 
      | SpiGeneral/SpiPhyUnit_0/SpiPhyUnitAsyncUseDma - false 
      | SpiGeneral/SpiPhyUnit_0/SpiPhyUnitClockRef - /Mcu/Mcu/McuModuleConfiguration/McuClockSettingConfig_0/SPI_CLK 
      | SpiGeneral/SpiPhyUnit_0/SpiPhyUnitMapping - LPSPI_2 
      | SpiGeneral/SpiPhyUnit_0/SpiPhyUnitMode - SPI_MASTER 
      | SpiGeneral/SpiPhyUnit_0/SpiPinConfiguration - 0 
      | SpiGeneral/SpiPhyUnit_0/SpiSamplePoint - 0 
      | SpiGeneral/SpiPhyUnit_1/Index - 1 
      | SpiGeneral/SpiPhyUnit_1/SpiPhyUnitAsyncUseDma - false 
      | SpiGeneral/SpiPhyUnit_1/SpiPhyUnitClockRef - /Mcu/Mcu/McuModuleConfiguration/McuClockSettingConfig_0/SPI_CLK 
      | SpiGeneral/SpiPhyUnit_1/SpiPhyUnitMapping - LPSPI_1 
      | SpiGeneral/SpiPhyUnit_1/SpiPhyUnitMode - SPI_SLAVE 
      | SpiGeneral/SpiPhyUnit_1/SpiPinConfiguration - 0 
      | SpiGeneral/SpiPhyUnit_1/SpiSamplePoint - 0 
      | SpiGeneral/SpiSupportConcurrentSyncTransmit - false 
      | SpiGeneral/SpiTimeoutMethod - OSIF_COUNTER_DUMMY 
      | SpiGeneral/SpiTransmitTimeout - 50000 
      | SpiGeneral/SpiVersionInfoApi - true 
      | SpiPublishedInformation - SpiPublishedInformation 
      | SpiPublishedInformation/SpiMaxHwUnit - 0
      settings: SourceProductName=ISR Blocks
  Terminator{1..7,9..13} (12 alike) : Terminator
  Triggered Subsystem12 : Subsystem  TreatAsAtomicUnit=on
  wires:
  Hardware_Interrupt_Handler/6 -> Terminator7/1
  Demux3/3 -> Terminator9/1
  Slave Interrupt Callback/2 -> Terminator3/1
  Hardware_Interrupt_Handler/4 -> Terminator4/1
  Hardware_Interrupt_Handler/2 -> Data Store Write37/1
  Hardware_Interrupt_Handler/1 -> Triggered Subsystem12/Trigger
  Demux3/8 -> Data Store Write38/1
  Demux3/2 -> Terminator5/1
  Hardware_Interrupt_Handler/3 -> Terminator1/1
  Slave Interrupt Callback/1 -> Function-Call Subsystem3/Trigger
  Demux3/1 -> Terminator2/1
  Demux3/6 -> Terminator12/1
  Demux3/5 -> Terminator11/1
  Master Interrupt Callback/1 -> Function-Call Subsystem1/Trigger
  Master Interrupt Callback/2 -> Terminator6/1
  Demux3/7 -> Terminator13/1
  Hardware_Interrupt_Handler/5 -> Demux3/1
  Demux3/4 -> Terminator10/1
